simulink model "AcasXuClosedLoop_all_2017b"  (Simulink 9.0)
settings: Solver=VariableStepAuto  StartTime=0.0  StopTime=20  RelTol=1e-3
libraries: neural x14040, simulink x2
(part 16 of 534)

== system AcasXuClosedLoop_all_2017b/networks/Networks_m_3/AcasXu_4_3/Layer 5 ==
  "a{4} " : Inport  PortDimensions=50
      mask Display: e = 0.06;c1 = [120 230 180]/255;c2 = ([120 230 180]+0)/(256+128);c3 = ([120 230 180]+128)/(256+128);patch([0 1 1 0],[0 0 1 1],c1);patch([0 1 1 1-e 1-e 0+e],[0 0 1 1-e 0+e 0+e],c2);patch([0 0 1 1-e 0+e 0+e],[0 1 1 1-e 1-e 0+e],c3);plot([0 1 1 0 0],[0 0 1 1 0]);
  Delays 1 : Subsystem
      mask Display: e = 0.06;c1 = [120 150 230]/255;c2 = ([120 150 230]+0)/383;c3 = ([120 150 230]+128)/383;patch([0 1 1 0],[0 0 1 1],c1);patch([0 1 1 1-e 1-e 0+e],[0 0 1 1-e 0+e 0+e],c2);patch([0 0 1 1-e 0+e 0+e],[0 1 1 1-e 1-e 0+e],c3);plot([0 1 1 0 0],[0 0 1 1 0]);disp('0');
  "LW{5,4}" : Subsystem
      mask Display: e = 0.06;c1 = [120 150 230]/255;c2 = ([120 150 230]+0)/383;c3 = ([120 150 230]+128)/383;patch([0 1 1 0],[0 0 1 1],c1);patch([0 1 1 1-e 1-e 0+e],[0 0 1 1-e 0+e 0+e],c2);patch([0 0 1 1-e 0+e 0+e],[0 1 1 1-e 1-e 0+e],c3);plot([0 1 1 0 0],[0 0 1 1 0]);disp('W');
  "b{5}" : Constant  Value=[2.711069999999999868833810978685505688190460205078125;0.373777000000000025892177291098050773143768310546875;0.57107300000000005280043069433304481208324432373046875;0.33265299999999997648814087369828484952449798583984375;-1.344160000000000021458390619955025613307952880859375;-2.043769999999999864570554564124904572963714599609375;0.38722800000000001663380544414394535124301910400390625;-2.6194199999999998595967554138042032718658447265625;1.088109999999999910613723841379396617412567138671875;-1.0533799999999999830180286153336055576801300048828125;-0.33796199999999998464517148022423498332500457763671875;0.9890689999999999759694446765934117138385772705078125;1.7318000000000000060396132539608515799045562744140625;-2.3428100000000000591171556152403354644775390625;0.63078599999999995784349948735325597226619720458984375;0.286934000000000022367885321727953851222991943359375;1.1716200000000001057998133546789176762104034423828125;-1.4532000000000000472510919280466623604297637939453125;1.1512299999999999755573298898525536060333251953125;0.352835999999999982978948764866800047457218170166015625;0.69320499999999996010302538707037456333637237548828125;0.9155189999999999717061882620328105986118316650390625;-0.073791200000000001235633817486814223229885101318359375;-0.701424999999999965183405947755090892314910888671875;-0.0099617400000000001780708913656781078316271305084228515625;0.67791400000000001657696202528313733637332916259765625;-0.375217999999999995974775401919032447040081024169921875;-3.0377700000000000812860889709554612636566162109375;-1.4288600000000000189714910447946749627590179443359375;-3.927169999999999827622332304599694907665252685546875;1.66561000000000003495870259939692914485931396484375;0.90458000000000005069722419648314826190471649169921875;0.284909000000000023344881583398091606795787811279296875;0.034817300000000002413447219851150293834507465362548828125;1.1870600000000000040500935938325710594654083251953125;-2.08082000000000011397105481592006981372833251953125;0.1708320000000000116191500865170382894575595855712890625;2.019769999999999843254272491321898996829986572265625;-1.8944199999999999928235183688229881227016448974609375;-0.045120100000000003259703618141429615207016468048095703125;1.4280699999999999505462255910970270633697509765625;0.7461349999999999926814098216709680855274200439453125;-1.094349999999999933919525574310682713985443115234375;-0.95453500000000002234656903965515084564685821533203125;0.27985599999999999365485336966230534017086029052734375;-1.14261999999999996902033672085963189601898193359375;0.34124100000000001653432946113753132522106170654296875;-1.4796300000000000007815970093361102044582366943359375;-0.210944999999999993622878946553100831806659698486328125;-1.10081000000000006622258297284133732318878173828125]
      mask Display: e = 0.06;c1 = [120 150 230]/255;c2 = ([120 150 230]+0)/383;c3 = ([120 150 230]+128)/383;patch([0 1 1 0],[0 0 1 1],c1);patch([0 1 1 1-e 1-e 0+e],[0 0 1 1-e 0+e 0+e],c2);patch([0 0 1 1-e 0+e 0+e],[0 1 1 1-e 1-e 0+e],c3);plot([0 1 1 0 0],[0 0 1 1 0]);disp('b');
  netsum : Sum  Inputs=2  SaturateOnIntegerOverflow=on  InputSameDT=on  OutDataTypeStr=Inherit: Same as first input
      mask Display: e = 0.06;c1 = [120 150 230]/255;c2 = ([120 150 230]+0)/383;c3 = ([120 150 230]+128)/383;patch([0 1 1 0],[0 0 1 1],c1);patch([0 1 1 1-e 1-e 0+e],[0 0 1 1-e 0+e 0+e],c2);patch([0 0 1 1-e 0+e 0+e],[0 1 1 1-e 1-e 0+e],c3);plot([0 1 1 0 0],[0 0 1 1 0]);disp('+');
  poslin : neural/Transfer Functions/poslin
      settings: SourceProductName=Deep Learning Toolbox  SourceProductBaseCode=NN
  "a{5}" : Outport  InitialOutput=[0;0;0;0;0;0;0;0;0;0;0;0;0;0;0;0;0;0;0;0;0;0;0;0;0;0;0;0;0;0;0;0;0;0;0;0;0;0;0;0;0;0;0;0;0;0;0;0;0;0]
      mask Display: e = 0.06;c1 = [120 230 180]/255;c2 = ([120 230 180]+0)/(256+128);c3 = ([120 230 180]+128)/(256+128);patch([0 1 1 0],[0 0 1 1],c1);patch([0 1 1 1-e 1-e 0+e],[0 0 1 1-e 0+e 0+e],c2);patch([0 0 1 1-e 0+e 0+e],[0 1 1 1-e 1-e 0+e],c3);plot([0 1 1 0 0],[0 0 1 1 0]);
  wires:
  poslin/1 -> "a{5}"/1
  netsum/1 -> poslin/1
  "b{5}"/1 -> netsum/2
  "LW{5,4}"/1("lz{5,4}") -> netsum/1
  Delays 1/1("pd{5,4}") -> "LW{5,4}"/1("ad{5,4}")
  "a{4} "/1 -> Delays 1/1("p{5}")

== system AcasXuClosedLoop_all_2017b/networks/Networks_m_3/AcasXu_4_3/Layer 6 ==
  "a{5} " : Inport  PortDimensions=50
      mask Display: e = 0.06;c1 = [120 230 180]/255;c2 = ([120 230 180]+0)/(256+128);c3 = ([120 230 180]+128)/(256+128);patch([0 1 1 0],[0 0 1 1],c1);patch([0 1 1 1-e 1-e 0+e],[0 0 1 1-e 0+e 0+e],c2);patch([0 0 1 1-e 0+e 0+e],[0 1 1 1-e 1-e 0+e],c3);plot([0 1 1 0 0],[0 0 1 1 0]);
  Delays 1 : Subsystem
      mask Display: e = 0.06;c1 = [120 150 230]/255;c2 = ([120 150 230]+0)/383;c3 = ([120 150 230]+128)/383;patch([0 1 1 0],[0 0 1 1],c1);patch([0 1 1 1-e 1-e 0+e],[0 0 1 1-e 0+e 0+e],c2);patch([0 0 1 1-e 0+e 0+e],[0 1 1 1-e 1-e 0+e],c3);plot([0 1 1 0 0],[0 0 1 1 0]);disp('0');
  "LW{6,5}" : Subsystem
      mask Display: e = 0.06;c1 = [120 150 230]/255;c2 = ([120 150 230]+0)/383;c3 = ([120 150 230]+128)/383;patch([0 1 1 0],[0 0 1 1],c1);patch([0 1 1 1-e 1-e 0+e],[0 0 1 1-e 0+e 0+e],c2);patch([0 0 1 1-e 0+e 0+e],[0 1 1 1-e 1-e 0+e],c3);plot([0 1 1 0 0],[0 0 1 1 0]);disp('W');
  "b{6}" : Constant  Value=[0.390301999999999982282616883821901865303516387939453125;0.03514219999999999843875997385111986659467220306396484375;0.48808499999999999108268866621074266731739044189453125;-0.78509200000000001207212108056410215795040130615234375;0.40505000000000002113864638886298052966594696044921875;0.354125999999999996337152197156683541834354400634765625;-2.48092999999999985760723575367592275142669677734375;0.48545300000000002338396143386489711701869964599609375;0.06425619999999999942819073339705937542021274566650390625;-0.66102099999999996970956317454692907631397247314453125;0.00843759000000000002061906201333840726874768733978271484375;-0.0248394000000000010619505275144547340460121631622314453125;0.278932000000000013262280162962269969284534454345703125;-0.11266500000000000125677246387567720375955104827880859375;0.5741709999999999869402245167293585836887359619140625;0.036816700000000000814193157339104800485074520111083984375;0.35369200000000000638777919448330067098140716552734375;1.1549300000000000121502807814977131783962249755859375;0.2562780000000000057980287238024175167083740234375;0.457390000000000018776091792460647411644458770751953125;0.10435600000000000431299440606380812823772430419921875;0.57056099999999998484412344623706303536891937255859375;0.8967899999999999760547098048846237361431121826171875;-0.03158829999999999971205255633321939967572689056396484375;-0.62138700000000002265920784338959492743015289306640625;0.385780000000000011795009413617663085460662841796875;0.234605000000000007975842208907124586403369903564453125;1.011810000000000098197006082045845687389373779296875;-0.169279000000000012793321957360603846609592437744140625;0.2365170000000000050111026439481065608561038970947265625;-0.4282369999999999787831939102034084498882293701171875;-0.55425899999999994616217691145720891654491424560546875;0.354545999999999972285280591677292250096797943115234375;-0.01203309999999999969910735586609007441438734531402587890625;0.61969700000000005335465402822592295706272125244140625;0.279428999999999982950527055436396040022373199462890625;-1.2366299999999998959765434847213327884674072265625;-0.36774099999999998455990635193302296102046966552734375;0.1561099999999999987654319966168259270489215850830078125;-0.256284000000000011798562127296463586390018463134765625;-0.01161180000000000024640289808530724258162081241607666015625;0.42765500000000000735411731511703692376613616943359375;-0.281951000000000007172928917498211376368999481201171875;0.33602100000000001411848415955319069325923919677734375;0.050025199999999998834443459827525657601654529571533203125;0.223339000000000009737988193592173047363758087158203125;0.4687769999999999992468247000942938029766082763671875;-1.3342400000000000925837184695410542190074920654296875;0.1654650000000000009681144774731365032494068145751953125;0.194346999999999991981525226947269402444362640380859375]
      mask Display: e = 0.06;c1 = [120 150 230]/255;c2 = ([120 150 230]+0)/383;c3 = ([120 150 230]+128)/383;patch([0 1 1 0],[0 0 1 1],c1);patch([0 1 1 1-e 1-e 0+e],[0 0 1 1-e 0+e 0+e],c2);patch([0 0 1 1-e 0+e 0+e],[0 1 1 1-e 1-e 0+e],c3);plot([0 1 1 0 0],[0 0 1 1 0]);disp('b');
  netsum : Sum  Inputs=2  SaturateOnIntegerOverflow=on  InputSameDT=on  OutDataTypeStr=Inherit: Same as first input
      mask Display: e = 0.06;c1 = [120 150 230]/255;c2 = ([120 150 230]+0)/383;c3 = ([120 150 230]+128)/383;patch([0 1 1 0],[0 0 1 1],c1);patch([0 1 1 1-e 1-e 0+e],[0 0 1 1-e 0+e 0+e],c2);patch([0 0 1 1-e 0+e 0+e],[0 1 1 1-e 1-e 0+e],c3);plot([0 1 1 0 0],[0 0 1 1 0]);disp('+');
  poslin : neural/Transfer Functions/poslin
      settings: SourceProductName=Deep Learning Toolbox  SourceProductBaseCode=NN
  "a{6}" : Outport  InitialOutput=[0;0;0;0;0;0;0;0;0;0;0;0;0;0;0;0;0;0;0;0;0;0;0;0;0;0;0;0;0;0;0;0;0;0;0;0;0;0;0;0;0;0;0;0;0;0;0;0;0;0]
      mask Display: e = 0.06;c1 = [120 230 180]/255;c2 = ([120 230 180]+0)/(256+128);c3 = ([120 230 180]+128)/(256+128);patch([0 1 1 0],[0 0 1 1],c1);patch([0 1 1 1-e 1-e 0+e],[0 0 1 1-e 0+e 0+e],c2);patch([0 0 1 1-e 0+e 0+e],[0 1 1 1-e 1-e 0+e],c3);plot([0 1 1 0 0],[0 0 1 1 0]);
  wires:
  poslin/1 -> "a{6}"/1
  netsum/1 -> poslin/1
  "b{6}"/1 -> netsum/2
  "LW{6,5}"/1("lz{6,5}") -> netsum/1
  Delays 1/1("pd{6,5}") -> "LW{6,5}"/1("ad{6,5}")
  "a{5} "/1 -> Delays 1/1("p{6}")

== system AcasXuClosedLoop_all_2017b/networks/Networks_m_3/AcasXu_4_3/Layer 7 ==
  "a{6} " : Inport  PortDimensions=50
      mask Display: e = 0.06;c1 = [120 230 180]/255;c2 = ([120 230 180]+0)/(256+128);c3 = ([120 230 180]+128)/(256+128);patch([0 1 1 0],[0 0 1 1],c1);patch([0 1 1 1-e 1-e 0+e],[0 0 1 1-e 0+e 0+e],c2);patch([0 0 1 1-e 0+e 0+e],[0 1 1 1-e 1-e 0+e],c3);plot([0 1 1 0 0],[0 0 1 1 0]);
  Delays 1 : Subsystem
      mask Display: e = 0.06;c1 = [120 150 230]/255;c2 = ([120 150 230]+0)/383;c3 = ([120 150 230]+128)/383;patch([0 1 1 0],[0 0 1 1],c1);patch([0 1 1 1-e 1-e 0+e],[0 0 1 1-e 0+e 0+e],c2);patch([0 0 1 1-e 0+e 0+e],[0 1 1 1-e 1-e 0+e],c3);plot([0 1 1 0 0],[0 0 1 1 0]);disp('0');
  "LW{7,6}" : Subsystem
      mask Display: e = 0.06;c1 = [120 150 230]/255;c2 = ([120 150 230]+0)/383;c3 = ([120 150 230]+128)/383;patch([0 1 1 0],[0 0 1 1],c1);patch([0 1 1 1-e 1-e 0+e],[0 0 1 1-e 0+e 0+e],c2);patch([0 0 1 1-e 0+e 0+e],[0 1 1 1-e 1-e 0+e],c3);plot([0 1 1 0 0],[0 0 1 1 0]);disp('W');
  "b{7}" : Constant  Value=[-0.0204165000000000006197264923457623808644711971282958984375;-0.019061399999999999177457965515714022330939769744873046875;0.01847669999999999868034450400955392979085445404052734375;-0.0185021999999999998964828051839504041709005832672119140625;0.01933920000000000083417717178235761821269989013671875]
      mask Display: e = 0.06;c1 = [120 150 230]/255;c2 = ([120 150 230]+0)/383;c3 = ([120 150 230]+128)/383;patch([0 1 1 0],[0 0 1 1],c1);patch([0 1 1 1-e 1-e 0+e],[0 0 1 1-e 0+e 0+e],c2);patch([0 0 1 1-e 0+e 0+e],[0 1 1 1-e 1-e 0+e],c3);plot([0 1 1 0 0],[0 0 1 1 0]);disp('b');
  netsum : Sum  Inputs=2  SaturateOnIntegerOverflow=on  InputSameDT=on  OutDataTypeStr=Inherit: Same as first input
      mask Display: e = 0.06;c1 = [120 150 230]/255;c2 = ([120 150 230]+0)/383;c3 = ([120 150 230]+128)/383;patch([0 1 1 0],[0 0 1 1],c1);patch([0 1 1 1-e 1-e 0+e],[0 0 1 1-e 0+e 0+e],c2);patch([0 0 1 1-e 0+e 0+e],[0 1 1 1-e 1-e 0+e],c3);plot([0 1 1 0 0],[0 0 1 1 0]);disp('+');
  purelin : neural/Transfer Functions/purelin
      settings: SourceProductName=Deep Learning Toolbox
  "a{7}" : Outport  InitialOutput=[0;0;0;0;0]
      mask Display: e = 0.06;c1 = [120 230 180]/255;c2 = ([120 230 180]+0)/(256+128);c3 = ([120 230 180]+128)/(256+128);patch([0 1 1 0],[0 0 1 1],c1);patch([0 1 1 1-e 1-e 0+e],[0 0 1 1-e 0+e 0+e],c2);patch([0 0 1 1-e 0+e 0+e],[0 1 1 1-e 1-e 0+e],c3);plot([0 1 1 0 0],[0 0 1 1 0]);
  wires:
  purelin/1 -> "a{7}"/1
  netsum/1 -> purelin/1
  "b{7}"/1 -> netsum/2
  "LW{7,6}"/1("lz{7,6}") -> netsum/1
  Delays 1/1("pd{7,6}") -> "LW{7,6}"/1("ad{7,6}")
  "a{6} "/1 -> Delays 1/1("p{7}")

== system AcasXuClosedLoop_all_2017b/networks/Networks_m_3/AcasXu_4_3/Process Input 1 ==
  x : Inport  PortDimensions=5
      mask Display: e = 0.06;c1 = [120 230 180]/255;c2 = ([120 230 180]+0)/(256+128);c3 = ([120 230 180]+128)/(256+128);patch([0 1 1 0],[0 0 1 1],c1);patch([0 1 1 1-e 1-e 0+e],[0 0 1 1-e 0+e 0+e],c2);patch([0 0 1 1-e 0+e 0+e],[0 1 1 1-e 1-e 0+e],c3);plot([0 1 1 0 0],[0 0 1 1 0]);
  p : Outport  PortDimensions=5
      mask Display: e = 0.06;c1 = [120 230 180]/255;c2 = ([120 230 180]+0)/(256+128);c3 = ([120 230 180]+128)/(256+128);patch([0 1 1 0],[0 0 1 1],c1);patch([0 1 1 1-e 1-e 0+e],[0 0 1 1-e 0+e 0+e],c2);patch([0 0 1 1-e 0+e 0+e],[0 1 1 1-e 1-e 0+e],c3);plot([0 1 1 0 0],[0 0 1 1 0]);
  wires:
  x/1 -> p/1

== system AcasXuClosedLoop_all_2017b/networks/Networks_m_3/AcasXu_4_3/Process Output 1 ==
  a : Inport  PortDimensions=5
      mask Display: e = 0.06;c1 = [120 230 180]/255;c2 = ([120 230 180]+0)/(256+128);c3 = ([120 230 180]+128)/(256+128);patch([0 1 1 0],[0 0 1 1],c1);patch([0 1 1 1-e 1-e 0+e],[0 0 1 1-e 0+e 0+e],c2);patch([0 0 1 1-e 0+e 0+e],[0 1 1 1-e 1-e 0+e],c3);plot([0 1 1 0 0],[0 0 1 1 0]);
  y : Outport  PortDimensions=5
      mask Display: e = 0.06;c1 = [120 230 180]/255;c2 = ([120 230 180]+0)/(256+128);c3 = ([120 230 180]+128)/(256+128);patch([0 1 1 0],[0 0 1 1],c1);patch([0 1 1 1-e 1-e 0+e],[0 0 1 1-e 0+e 0+e],c2);patch([0 0 1 1-e 0+e 0+e],[0 1 1 1-e 1-e 0+e],c3);plot([0 1 1 0 0],[0 0 1 1 0]);
  wires:
  a/1 -> y/1

== system AcasXuClosedLoop_all_2017b/networks/Networks_m_3/AcasXu_5_3/Layer 1 ==
  "p{1}" : Inport  PortDimensions=5
      mask Display: e = 0.06;c1 = [120 230 180]/255;c2 = ([120 230 180]+0)/(256+128);c3 = ([120 230 180]+128)/(256+128);patch([0 1 1 0],[0 0 1 1],c1);patch([0 1 1 1-e 1-e 0+e],[0 0 1 1-e 0+e 0+e],c2);patch([0 0 1 1-e 0+e 0+e],[0 1 1 1-e 1-e 0+e],c3);plot([0 1 1 0 0],[0 0 1 1 0]);
  Delays 1 : Subsystem
      mask Display: e = 0.06;c1 = [120 150 230]/255;c2 = ([120 150 230]+0)/383;c3 = ([120 150 230]+128)/383;patch([0 1 1 0],[0 0 1 1],c1);patch([0 1 1 1-e 1-e 0+e],[0 0 1 1-e 0+e 0+e],c2);patch([0 0 1 1-e 0+e 0+e],[0 1 1 1-e 1-e 0+e],c3);plot([0 1 1 0 0],[0 0 1 1 0]);disp('0');
  "IW{1,1}" : Subsystem
      mask Display: e = 0.06;c1 = [120 150 230]/255;c2 = ([120 150 230]+0)/383;c3 = ([120 150 230]+128)/383;patch([0 1 1 0],[0 0 1 1],c1);patch([0 1 1 1-e 1-e 0+e],[0 0 1 1-e 0+e 0+e],c2);patch([0 0 1 1-e 0+e 0+e],[0 1 1 1-e 1-e 0+e],c3);plot([0 1 1 0 0],[0 0 1 1 0]);disp('W');
  "b{1}" : Constant  Value=[-0.3715450000000000141398004416259936988353729248046875;0.65812199999999998478728002737625502049922943115234375;-0.2231499999999999872546396773032029159367084503173828125;0.52022299999999999098321268320432864129543304443359375;0.0173735999999999994602983832692189025692641735076904296875;-0.03446559999999999901465486118468106724321842193603515625;0.1578150000000000108268949361445265822112560272216796875;-0.299049999999999982502885131907532922923564910888671875;-0.672749000000000041410430640098638832569122314453125;-0.64948200000000000375877107217092998325824737548828125;-0.50798299999999996234834043207229115068912506103515625;-0.69301800000000002288658151883282698690891265869140625;0.060070400000000002960209855018547386862337589263916015625;-0.12440199999999999869171318778171553276479244232177734375;-0.387757999999999991569410440206411294639110565185546875;-0.007049680000000000008875122858853501384146511554718017578125;0.384906000000000025895730004776851274073123931884765625;-0.060446699999999999042277210037354961968958377838134765625;-0.83811400000000002563638190622441470623016357421875;0.183143000000000000238031816479633562266826629638671875;0.0108234000000000003816058580241588060744106769561767578125;-0.489862999999999992883914501362596638500690460205078125;-0.88090999999999997083222069704788736999034881591796875;0.42101100000000002410160959698259830474853515625;0.000578735000000000034993397068916465286747552454471588134765625;-0.352814000000000016488144183313124813139438629150390625;-0.054441200000000002090505546448184759356081485748291015625;0.016837600000000001176925223944635945372283458709716796875;-0.019340400000000000646505071699721156619489192962646484375;0.2896549999999999958077978590154089033603668212890625;-0.48292000000000001591615728102624416351318359375;-0.5773070000000000145945477925124578177928924560546875;-0.0178104000000000005365929922618306591175496578216552734375;-0.66247000000000000330402372128446586430072784423828125;-0.0340055999999999969407582511848886497318744659423828125;-0.222245999999999999108268866621074266731739044189453125;-0.12642900000000001359268253509071655571460723876953125;-0.420171000000000016694201576683553867042064666748046875;0.235551000000000010370371228418662212789058685302734375;0.0307842000000000011461498417020266060717403888702392578125;0.213629000000000013326229009180678986012935638427734375;-0.0248257999999999985629717258461823803372681140899658203125;0.090437500000000003996802888650563545525074005126953125;0.251244999999999996109778521713451482355594635009765625;0.305526000000000019785062477239989675581455230712890625;-0.12407100000000000072919448257380281575024127960205078125;-0.0225280999999999988203658318752786726690828800201416015625;-0.2296080000000000065352168121535214595496654510498046875;0.12387600000000000000088817841970012523233890533447265625;0.1450490000000000112567732912793871946632862091064453125]
      mask Display: e = 0.06;c1 = [120 150 230]/255;c2 = ([120 150 230]+0)/383;c3 = ([120 150 230]+128)/383;patch([0 1 1 0],[0 0 1 1],c1);patch([0 1 1 1-e 1-e 0+e],[0 0 1 1-e 0+e 0+e],c2);patch([0 0 1 1-e 0+e 0+e],[0 1 1 1-e 1-e 0+e],c3);plot([0 1 1 0 0],[0 0 1 1 0]);disp('b');
  netsum : Sum  Inputs=2  SaturateOnIntegerOverflow=on  InputSameDT=on  OutDataTypeStr=Inherit: Same as first input
      mask Display: e = 0.06;c1 = [120 150 230]/255;c2 = ([120 150 230]+0)/383;c3 = ([120 150 230]+128)/383;patch([0 1 1 0],[0 0 1 1],c1);patch([0 1 1 1-e 1-e 0+e],[0 0 1 1-e 0+e 0+e],c2);patch([0 0 1 1-e 0+e 0+e],[0 1 1 1-e 1-e 0+e],c3);plot([0 1 1 0 0],[0 0 1 1 0]);disp('+');
  poslin : neural/Transfer Functions/poslin
      settings: SourceProductName=Deep Learning Toolbox  SourceProductBaseCode=NN
  "a{1}" : Outport  InitialOutput=[0;0;0;0;0;0;0;0;0;0;0;0;0;0;0;0;0;0;0;0;0;0;0;0;0;0;0;0;0;0;0;0;0;0;0;0;0;0;0;0;0;0;0;0;0;0;0;0;0;0]
      mask Display: e = 0.06;c1 = [120 230 180]/255;c2 = ([120 230 180]+0)/(256+128);c3 = ([120 230 180]+128)/(256+128);patch([0 1 1 0],[0 0 1 1],c1);patch([0 1 1 1-e 1-e 0+e],[0 0 1 1-e 0+e 0+e],c2);patch([0 0 1 1-e 0+e 0+e],[0 1 1 1-e 1-e 0+e],c3);plot([0 1 1 0 0],[0 0 1 1 0]);
  wires:
  poslin/1 -> "a{1}"/1
  netsum/1 -> poslin/1
  "b{1}"/1 -> netsum/2
  "IW{1,1}"/1("iz{1,1}") -> netsum/1
  Delays 1/1("pd{1,1}") -> "IW{1,1}"/1("pd{1,1}")
  "p{1}"/1 -> Delays 1/1("p{1}")

== system AcasXuClosedLoop_all_2017b/networks/Networks_m_3/AcasXu_5_3/Layer 2 ==
  "a{1} " : Inport  PortDimensions=50
      mask Display: e = 0.06;c1 = [120 230 180]/255;c2 = ([120 230 180]+0)/(256+128);c3 = ([120 230 180]+128)/(256+128);patch([0 1 1 0],[0 0 1 1],c1);patch([0 1 1 1-e 1-e 0+e],[0 0 1 1-e 0+e 0+e],c2);patch([0 0 1 1-e 0+e 0+e],[0 1 1 1-e 1-e 0+e],c3);plot([0 1 1 0 0],[0 0 1 1 0]);
  Delays 1 : Subsystem
      mask Display: e = 0.06;c1 = [120 150 230]/255;c2 = ([120 150 230]+0)/383;c3 = ([120 150 230]+128)/383;patch([0 1 1 0],[0 0 1 1],c1);patch([0 1 1 1-e 1-e 0+e],[0 0 1 1-e 0+e 0+e],c2);patch([0 0 1 1-e 0+e 0+e],[0 1 1 1-e 1-e 0+e],c3);plot([0 1 1 0 0],[0 0 1 1 0]);disp('0');
  "LW{2,1}" : Subsystem
      mask Display: e = 0.06;c1 = [120 150 230]/255;c2 = ([120 150 230]+0)/383;c3 = ([120 150 230]+128)/383;patch([0 1 1 0],[0 0 1 1],c1);patch([0 1 1 1-e 1-e 0+e],[0 0 1 1-e 0+e 0+e],c2);patch([0 0 1 1-e 0+e 0+e],[0 1 1 1-e 1-e 0+e],c3);plot([0 1 1 0 0],[0 0 1 1 0]);disp('W');
  "b{2}" : Constant  Value=[-0.1676829999999999987192467187924194149672985076904296875;0.310601999999999989210408557482878677546977996826171875;-0.96966600000000002790301323329913429915904998779296875;-0.041570500000000003282263350001812796108424663543701171875;-0.481177000000000021362467350627412088215351104736328125;-0.65698599999999995890931359099340625107288360595703125;-0.06350719999999999976214581920430646277964115142822265625;0.0496308000000000026918911544271395541727542877197265625;1.4830300000000000704147851138259284198284149169921875;-0.347907000000000021788792992083472199738025665283203125;-0.007282730000000000279702927485914187855087220668792724609375;0.3084700000000000219557705349870957434177398681640625;1.3338699999999998890842789478483609855175018310546875;0.4662450000000000205346850634668953716754913330078125;-0.01525540000000000055713211821739605511538684368133544921875;-0.50505999999999995342392367092543281614780426025390625;-0.43938500000000002554401135057560168206691741943359375;-0.73899400000000003974065521106240339577198028564453125;0.65507499999999996287414205653476528823375701904296875;-0.164113000000000008871126055964850820600986480712890625;-0.05797120000000000050732751333271153271198272705078125;1.4484500000000000152766688188421539962291717529296875;0.020181100000000000427302637717730249278247356414794921875;-0.4089059999999999917008608463220298290252685546875;-0.37971100000000002072653160212212242186069488525390625;-0.70986000000000004650502205549855716526508331298828125;-0.95234399999999996833111026717233471572399139404296875;-0.017702099999999998392041788974893279373645782470703125;-1.8702300000000000590461013416643254458904266357421875;-0.00840982000000000014250378654878659290261566638946533203125;0.0224244000000000005823341808763871085830032825469970703125;-0.6815130000000000354276608049985952675342559814453125;-0.5405290000000000372182284991140477359294891357421875;0.1988499999999999989785948173448559828102588653564453125;0.03694530000000000036219915955371106974780559539794921875;-0.492931999999999981287857053757761605083942413330078125;-0.01329559999999999943265382995605250471271574497222900390625;-0.0114491000000000002490008199629301088862121105194091796875;0.89915599999999995528554563861689530313014984130859375;-0.159463999999999994638955058690044097602367401123046875;-1.50035000000000007247535904753021895885467529296875;0.57026699999999996837374283131794072687625885009765625;-1.3336099999999999621280721839866600930690765380859375;-0.27888400000000002076916416626772843301296234130859375;-0.40673999999999999044320020402665250003337860107421875;-0.75057099999999998818367430430953390896320343017578125;0.62766299999999997094590753476950339972972869873046875;0.558486999999999955690554998000152409076690673828125;-0.0251526000000000006517897332969369017519056797027587890625;-0.060903300000000000491429119620079291053116321563720703125]
      mask Display: e = 0.06;c1 = [120 150 230]/255;c2 = ([120 150 230]+0)/383;c3 = ([120 150 230]+128)/383;patch([0 1 1 0],[0 0 1 1],c1);patch([0 1 1 1-e 1-e 0+e],[0 0 1 1-e 0+e 0+e],c2);patch([0 0 1 1-e 0+e 0+e],[0 1 1 1-e 1-e 0+e],c3);plot([0 1 1 0 0],[0 0 1 1 0]);disp('b');
  netsum : Sum  Inputs=2  SaturateOnIntegerOverflow=on  InputSameDT=on  OutDataTypeStr=Inherit: Same as first input
      mask Display: e = 0.06;c1 = [120 150 230]/255;c2 = ([120 150 230]+0)/383;c3 = ([120 150 230]+128)/383;patch([0 1 1 0],[0 0 1 1],c1);patch([0 1 1 1-e 1-e 0+e],[0 0 1 1-e 0+e 0+e],c2);patch([0 0 1 1-e 0+e 0+e],[0 1 1 1-e 1-e 0+e],c3);plot([0 1 1 0 0],[0 0 1 1 0]);disp('+');
  poslin : neural/Transfer Functions/poslin
      settings: SourceProductName=Deep Learning Toolbox  SourceProductBaseCode=NN
  "a{2}" : Outport  InitialOutput=[0;0;0;0;0;0;0;0;0;0;0;0;0;0;0;0;0;0;0;0;0;0;0;0;0;0;0;0;0;0;0;0;0;0;0;0;0;0;0;0;0;0;0;0;0;0;0;0;0;0]
      mask Display: e = 0.06;c1 = [120 230 180]/255;c2 = ([120 230 180]+0)/(256+128);c3 = ([120 230 180]+128)/(256+128);patch([0 1 1 0],[0 0 1 1],c1);patch([0 1 1 1-e 1-e 0+e],[0 0 1 1-e 0+e 0+e],c2);patch([0 0 1 1-e 0+e 0+e],[0 1 1 1-e 1-e 0+e],c3);plot([0 1 1 0 0],[0 0 1 1 0]);
  wires:
  poslin/1 -> "a{2}"/1
  netsum/1 -> poslin/1
  "b{2}"/1 -> netsum/2
  "LW{2,1}"/1("lz{2,1}") -> netsum/1
  Delays 1/1("pd{2,1}") -> "LW{2,1}"/1("ad{2,1}")
  "a{1} "/1 -> Delays 1/1("p{2}")

== system AcasXuClosedLoop_all_2017b/networks/Networks_m_3/AcasXu_5_3/Layer 3 ==
  "a{2} " : Inport  PortDimensions=50
      mask Display: e = 0.06;c1 = [120 230 180]/255;c2 = ([120 230 180]+0)/(256+128);c3 = ([120 230 180]+128)/(256+128);patch([0 1 1 0],[0 0 1 1],c1);patch([0 1 1 1-e 1-e 0+e],[0 0 1 1-e 0+e 0+e],c2);patch([0 0 1 1-e 0+e 0+e],[0 1 1 1-e 1-e 0+e],c3);plot([0 1 1 0 0],[0 0 1 1 0]);
  Delays 1 : Subsystem
      mask Display: e = 0.06;c1 = [120 150 230]/255;c2 = ([120 150 230]+0)/383;c3 = ([120 150 230]+128)/383;patch([0 1 1 0],[0 0 1 1],c1);patch([0 1 1 1-e 1-e 0+e],[0 0 1 1-e 0+e 0+e],c2);patch([0 0 1 1-e 0+e 0+e],[0 1 1 1-e 1-e 0+e],c3);plot([0 1 1 0 0],[0 0 1 1 0]);disp('0');
  "LW{3,2}" : Subsystem
      mask Display: e = 0.06;c1 = [120 150 230]/255;c2 = ([120 150 230]+0)/383;c3 = ([120 150 230]+128)/383;patch([0 1 1 0],[0 0 1 1],c1);patch([0 1 1 1-e 1-e 0+e],[0 0 1 1-e 0+e 0+e],c2);patch([0 0 1 1-e 0+e 0+e],[0 1 1 1-e 1-e 0+e],c3);plot([0 1 1 0 0],[0 0 1 1 0]);disp('W');
  "b{3}" : Constant  Value=[0.1510060000000000013375967000683885999023914337158203125;0.78885499999999997289279463075217790901660919189453125;-1.1981900000000000883204620549804531037807464599609375;-0.588840999999999947789319776347838342189788818359375;-0.397129000000000009773515330380178056657314300537109375;-0.280633999999999994567900785114034079015254974365234375;1.6851499999999999257482841130695305764675140380859375;-0.6019039999999999945856643535080365836620330810546875;-1.6417800000000000171240799318184144794940948486328125;-2.115330000000000154614099301397800445556640625;-0.0430941999999999991732835269431234337389469146728515625;-0.75815500000000002334132886971929110586643218994140625;-0.0785547000000000050778936611095559783279895782470703125;1.3500199999999999977973175191436894237995147705078125;-1.826869999999999993889332472463138401508331298828125;-1.043509999999999937614347800263203680515289306640625;-0.551185999999999953757878756732679903507232666015625;-2.163539999999999796642669025459326803684234619140625;-0.84310300000000004683187171394820325076580047607421875;-0.2149149999999999949285012235122849233448505401611328125;-0.8115930000000000088533624875708483159542083740234375;0.55653099999999999791810978422290645539760589599609375;0.55281100000000005234568334344658069312572479248046875;0.106677999999999995051069845430902205407619476318359375;0.48474899999999998545519019899074919521808624267578125;0.34542499999999998205879592205747030675411224365234375;1.12647999999999992581933838664554059505462646484375;-1.0468900000000000982680603556218557059764862060546875;-1.76700999999999996958877090946771204471588134765625;-0.141981999999999997097432924419990740716457366943359375;0.81875200000000003530686853991937823593616485595703125;-1.427729999999999943582906780648045241832733154296875;-0.88332200000000005157829718882567249238491058349609375;-0.58885799999999999254640670187654905021190643310546875;1.00330999999999992411403582082130014896392822265625;0.323604000000000002756905814749188721179962158203125;-2.16692999999999980076381689286790788173675537109375;0.34770800000000001706013108560000546276569366455078125;-0.61344100000000001404742988597718067467212677001953125;-3.37966999999999995196731106261722743511199951171875;-0.318205000000000015614176618328201584517955780029296875;-0.065882300000000004747136017613229341804981231689453125;0.2342640000000000000124344978758017532527446746826171875;-1.055919999999999969730879456619732081890106201171875;0.7741190000000000015489831639570184051990509033203125;0.44780399999999997984190258648595772683620452880859375;-0.554389000000000020662582755903713405132293701171875;-0.83642099999999997006483454242697916924953460693359375;1.2263699999999999601385525238583795726299285888671875;0.2497109999999999885300638879925827495753765106201171875]
      mask Display: e = 0.06;c1 = [120 150 230]/255;c2 = ([120 150 230]+0)/383;c3 = ([120 150 230]+128)/383;patch([0 1 1 0],[0 0 1 1],c1);patch([0 1 1 1-e 1-e 0+e],[0 0 1 1-e 0+e 0+e],c2);patch([0 0 1 1-e 0+e 0+e],[0 1 1 1-e 1-e 0+e],c3);plot([0 1 1 0 0],[0 0 1 1 0]);disp('b');
  netsum : Sum  Inputs=2  SaturateOnIntegerOverflow=on  InputSameDT=on  OutDataTypeStr=Inherit: Same as first input
      mask Display: e = 0.06;c1 = [120 150 230]/255;c2 = ([120 150 230]+0)/383;c3 = ([120 150 230]+128)/383;patch([0 1 1 0],[0 0 1 1],c1);patch([0 1 1 1-e 1-e 0+e],[0 0 1 1-e 0+e 0+e],c2);patch([0 0 1 1-e 0+e 0+e],[0 1 1 1-e 1-e 0+e],c3);plot([0 1 1 0 0],[0 0 1 1 0]);disp('+');
  poslin : neural/Transfer Functions/poslin
      settings: SourceProductName=Deep Learning Toolbox  SourceProductBaseCode=NN
  "a{3}" : Outport  InitialOutput=[0;0;0;0;0;0;0;0;0;0;0;0;0;0;0;0;0;0;0;0;0;0;0;0;0;0;0;0;0;0;0;0;0;0;0;0;0;0;0;0;0;0;0;0;0;0;0;0;0;0]
      mask Display: e = 0.06;c1 = [120 230 180]/255;c2 = ([120 230 180]+0)/(256+128);c3 = ([120 230 180]+128)/(256+128);patch([0 1 1 0],[0 0 1 1],c1);patch([0 1 1 1-e 1-e 0+e],[0 0 1 1-e 0+e 0+e],c2);patch([0 0 1 1-e 0+e 0+e],[0 1 1 1-e 1-e 0+e],c3);plot([0 1 1 0 0],[0 0 1 1 0]);
  wires:
  poslin/1 -> "a{3}"/1
  netsum/1 -> poslin/1
  "b{3}"/1 -> netsum/2
  "LW{3,2}"/1("lz{3,2}") -> netsum/1
  Delays 1/1("pd{3,2}") -> "LW{3,2}"/1("ad{3,2}")
  "a{2} "/1 -> Delays 1/1("p{3}")

== system AcasXuClosedLoop_all_2017b/networks/Networks_m_3/AcasXu_5_3/Layer 4 ==
  "a{3} " : Inport  PortDimensions=50
      mask Display: e = 0.06;c1 = [120 230 180]/255;c2 = ([120 230 180]+0)/(256+128);c3 = ([120 230 180]+128)/(256+128);patch([0 1 1 0],[0 0 1 1],c1);patch([0 1 1 1-e 1-e 0+e],[0 0 1 1-e 0+e 0+e],c2);patch([0 0 1 1-e 0+e 0+e],[0 1 1 1-e 1-e 0+e],c3);plot([0 1 1 0 0],[0 0 1 1 0]);
  Delays 1 : Subsystem
      mask Display: e = 0.06;c1 = [120 150 230]/255;c2 = ([120 150 230]+0)/383;c3 = ([120 150 230]+128)/383;patch([0 1 1 0],[0 0 1 1],c1);patch([0 1 1 1-e 1-e 0+e],[0 0 1 1-e 0+e 0+e],c2);patch([0 0 1 1-e 0+e 0+e],[0 1 1 1-e 1-e 0+e],c3);plot([0 1 1 0 0],[0 0 1 1 0]);disp('0');
  "LW{4,3}" : Subsystem
      mask Display: e = 0.06;c1 = [120 150 230]/255;c2 = ([120 150 230]+0)/383;c3 = ([120 150 230]+128)/383;patch([0 1 1 0],[0 0 1 1],c1);patch([0 1 1 1-e 1-e 0+e],[0 0 1 1-e 0+e 0+e],c2);patch([0 0 1 1-e 0+e 0+e],[0 1 1 1-e 1-e 0+e],c3);plot([0 1 1 0 0],[0 0 1 1 0]);disp('W');
  "b{4}" : Constant  Value=[0.7212579999999999547100060226512141525745391845703125;0.54630999999999996230570786792668513953685760498046875;1.1140399999999999192112909440766088664531707763671875;-0.442867999999999983895548893997329287230968475341796875;0.3276339999999999808011352797620929777622222900390625;0.08699379999999999613091716810231446288526058197021484375;-0.95715300000000003155520289510604925453662872314453125;0.2160539999999999960511587460132432170212268829345703125;-4.0011200000000002319211489520967006683349609375;-0.326593999999999995420552068026154302060604095458984375;-0.652746000000000048402171159978024661540985107421875;-0.023803100000000000646682707383661181665956974029541015625;-0.75029800000000002047073621724848635494709014892578125;0.06980310000000000680842049405327998101711273193359375;-0.1605459999999999942676964792553917504847049713134765625;-0.215036000000000004916955731459893286228179931640625;0.53674999999999994937383007709286175668239593505859375;0.496682000000000012374101743262144736945629119873046875;-1.4020900000000000584776671530562452971935272216796875;0.2827859999999999818243168192566372454166412353515625;0.305470999999999992535748560840147547423839569091796875;-0.70448699999999997434230181170278228819370269775390625;-0.80505300000000001858779796748422086238861083984375;1.288419999999999898676605880609713494777679443359375;0.2052150000000000085176310449242009781301021575927734375;-0.6115939999999999709956455262727104127407073974609375;-0.19087799999999999212008106042048893868923187255859375;-1.2522200000000001107736125049996189773082733154296875;-0.65776000000000001133315663537359796464443206787109375;0.0227152000000000013624212868990071001462638378143310546875;0.78801799999999999624122892782907001674175262451171875;0.99007400000000000961364321483415551483631134033203125;0.09256599999999999550670537473706644959747791290283203125;0.261581000000000007954525926834321580827236175537109375;-0.472055999999999975624831449749763123691082000732421875;-0.410337000000000007293721182577428407967090606689453125;-0.256960000000000021724844145865063183009624481201171875;0.52285400000000004094857786185457371175289154052734375;0.307966000000000017511325722807669080793857574462890625;-2.04220999999999985874410413089208304882049560546875;0.1452279999999999959836571861160336993634700775146484375;0.570680999999999993832489053602330386638641357421875;-1.0596300000000000718358705853461287915706634521484375;-0.64597499999999996589394868351519107818603515625;0.8822870000000000434425828643725253641605377197265625;-0.011801499999999999490629676301978179253637790679931640625;-0.16074800000000000199662508748588152229785919189453125;-0.51775300000000001876543365142424590885639190673828125;-3.15078000000000013613998817163519561290740966796875;0.30289500000000002533084852984757162630558013916015625]
      mask Display: e = 0.06;c1 = [120 150 230]/255;c2 = ([120 150 230]+0)/383;c3 = ([120 150 230]+128)/383;patch([0 1 1 0],[0 0 1 1],c1);patch([0 1 1 1-e 1-e 0+e],[0 0 1 1-e 0+e 0+e],c2);patch([0 0 1 1-e 0+e 0+e],[0 1 1 1-e 1-e 0+e],c3);plot([0 1 1 0 0],[0 0 1 1 0]);disp('b');
  netsum : Sum  Inputs=2  SaturateOnIntegerOverflow=on  InputSameDT=on  OutDataTypeStr=Inherit: Same as first input
      mask Display: e = 0.06;c1 = [120 150 230]/255;c2 = ([120 150 230]+0)/383;c3 = ([120 150 230]+128)/383;patch([0 1 1 0],[0 0 1 1],c1);patch([0 1 1 1-e 1-e 0+e],[0 0 1 1-e 0+e 0+e],c2);patch([0 0 1 1-e 0+e 0+e],[0 1 1 1-e 1-e 0+e],c3);plot([0 1 1 0 0],[0 0 1 1 0]);disp('+');
  poslin : neural/Transfer Functions/poslin
      settings: SourceProductName=Deep Learning Toolbox  SourceProductBaseCode=NN
  "a{4}" : Outport  InitialOutput=[0;0;0;0;0;0;0;0;0;0;0;0;0;0;0;0;0;0;0;0;0;0;0;0;0;0;0;0;0;0;0;0;0;0;0;0;0;0;0;0;0;0;0;0;0;0;0;0;0;0]
      mask Display: e = 0.06;c1 = [120 230 180]/255;c2 = ([120 230 180]+0)/(256+128);c3 = ([120 230 180]+128)/(256+128);patch([0 1 1 0],[0 0 1 1],c1);patch([0 1 1 1-e 1-e 0+e],[0 0 1 1-e 0+e 0+e],c2);patch([0 0 1 1-e 0+e 0+e],[0 1 1 1-e 1-e 0+e],c3);plot([0 1 1 0 0],[0 0 1 1 0]);
  wires:
  poslin/1 -> "a{4}"/1
  netsum/1 -> poslin/1
  "b{4}"/1 -> netsum/2
  "LW{4,3}"/1("lz{4,3}") -> netsum/1
  Delays 1/1("pd{4,3}") -> "LW{4,3}"/1("ad{4,3}")
  "a{3} "/1 -> Delays 1/1("p{4}")

== system AcasXuClosedLoop_all_2017b/networks/Networks_m_3/AcasXu_5_3/Layer 5 ==
  "a{4} " : Inport  PortDimensions=50
      mask Display: e = 0.06;c1 = [120 230 180]/255;c2 = ([120 230 180]+0)/(256+128);c3 = ([120 230 180]+128)/(256+128);patch([0 1 1 0],[0 0 1 1],c1);patch([0 1 1 1-e 1-e 0+e],[0 0 1 1-e 0+e 0+e],c2);patch([0 0 1 1-e 0+e 0+e],[0 1 1 1-e 1-e 0+e],c3);plot([0 1 1 0 0],[0 0 1 1 0]);
  Delays 1 : Subsystem
      mask Display: e = 0.06;c1 = [120 150 230]/255;c2 = ([120 150 230]+0)/383;c3 = ([120 150 230]+128)/383;patch([0 1 1 0],[0 0 1 1],c1);patch([0 1 1 1-e 1-e 0+e],[0 0 1 1-e 0+e 0+e],c2);patch([0 0 1 1-e 0+e 0+e],[0 1 1 1-e 1-e 0+e],c3);plot([0 1 1 0 0],[0 0 1 1 0]);disp('0');
  "LW{5,4}" : Subsystem
      mask Display: e = 0.06;c1 = [120 150 230]/255;c2 = ([120 150 230]+0)/383;c3 = ([120 150 230]+128)/383;patch([0 1 1 0],[0 0 1 1],c1);patch([0 1 1 1-e 1-e 0+e],[0 0 1 1-e 0+e 0+e],c2);patch([0 0 1 1-e 0+e 0+e],[0 1 1 1-e 1-e 0+e],c3);plot([0 1 1 0 0],[0 0 1 1 0]);disp('W');
  "b{5}" : Constant  Value=[0.47258400000000000407140987590537406504154205322265625;1.2492300000000000625988150204648263752460479736328125;-4.52592000000000016513013179064728319644927978515625;-0.6574619999999999908624204181251116096973419189453125;-0.028083400000000001417443939999429858289659023284912109375;-0.2020530000000000103721475852580624632537364959716796875;0.70148200000000004994404889657744206488132476806640625;0.6753489999999999771063130538095720112323760986328125;0.75429000000000001602273869139025919139385223388671875;-0.2459340000000000137081457296517328359186649322509765625;0.0716490999999999933489647219175822101533412933349609375;0.222434999999999993836041767281130887567996978759765625;-0.8294580000000000286064505417016334831714630126953125;-0.31864700000000001356426082566031254827976226806640625;-0.00776489999999999992719157404508223407901823520660400390625;0.945052000000000003154809746774844825267791748046875;-4.44582999999999994855670593096874654293060302734375;1.0560400000000000897415475265006534755229949951171875;1.6571499999999999008792883614660240709781646728515625;-0.8016609999999999569553210676531307399272918701171875;1.576729999999999964899188853451050817966461181640625;1.026149999999999895550217843265272676944732666015625;0.049465900000000000147348799828250776045024394989013671875;1.497759999999999980246911945869214832782745361328125;0.249765999999999988023802188763511367142200469970703125;-1.4057500000000000550670620214077644050121307373046875;-0.97891399999999995085175896747387014329433441162109375;-1.11186000000000007048583938740193843841552734375;0.63749500000000003385736135896877385675907135009765625;0.92279999999999995363708649165346287190914154052734375;0.77215100000000003177547114319168031215667724609375;1.1934899999999999398170302811195142567157745361328125;1.3931199999999999139532746994518674910068511962890625;0.484723999999999988208543300061137415468692779541015625;-1.9544799999999999950972551232553087174892425537109375;1.057889999999999997015720509807579219341278076171875;1.955319999999999946993511912296526134014129638671875;-0.8511889999999999734114908278570510447025299072265625;1.4870300000000000739674987926264293491840362548828125;-0.427566999999999974857445295128854922950267791748046875;0.601442000000000032144953365786932408809661865234375;0.38846999999999998198774164848146028816699981689453125;-1.3176200000000000134292577058658935129642486572265625;0.454407999999999978602005512584582902491092681884765625;-0.0168409999999999983322229724080898449756205081939697265625;-0.57140199999999996549604475148953497409820556640625;-1.84166000000000007474909580196253955364227294921875;1.2264999999999999236166559057892300188541412353515625;0.50610399999999999831601371624856255948543548583984375;-0.775908999999999959840124574839137494564056396484375]
      mask Display: e = 0.06;c1 = [120 150 230]/255;c2 = ([120 150 230]+0)/383;c3 = ([120 150 230]+128)/383;patch([0 1 1 0],[0 0 1 1],c1);patch([0 1 1 1-e 1-e 0+e],[0 0 1 1-e 0+e 0+e],c2);patch([0 0 1 1-e 0+e 0+e],[0 1 1 1-e 1-e 0+e],c3);plot([0 1 1 0 0],[0 0 1 1 0]);disp('b');
  netsum : Sum  Inputs=2  SaturateOnIntegerOverflow=on  InputSameDT=on  OutDataTypeStr=Inherit: Same as first input
      mask Display: e = 0.06;c1 = [120 150 230]/255;c2 = ([120 150 230]+0)/383;c3 = ([120 150 230]+128)/383;patch([0 1 1 0],[0 0 1 1],c1);patch([0 1 1 1-e 1-e 0+e],[0 0 1 1-e 0+e 0+e],c2);patch([0 0 1 1-e 0+e 0+e],[0 1 1 1-e 1-e 0+e],c3);plot([0 1 1 0 0],[0 0 1 1 0]);disp('+');
  poslin : neural/Transfer Functions/poslin
      settings: SourceProductName=Deep Learning Toolbox  SourceProductBaseCode=NN
  "a{5}" : Outport  InitialOutput=[0;0;0;0;0;0;0;0;0;0;0;0;0;0;0;0;0;0;0;0;0;0;0;0;0;0;0;0;0;0;0;0;0;0;0;0;0;0;0;0;0;0;0;0;0;0;0;0;0;0]
      mask Display: e = 0.06;c1 = [120 230 180]/255;c2 = ([120 230 180]+0)/(256+128);c3 = ([120 230 180]+128)/(256+128);patch([0 1 1 0],[0 0 1 1],c1);patch([0 1 1 1-e 1-e 0+e],[0 0 1 1-e 0+e 0+e],c2);patch([0 0 1 1-e 0+e 0+e],[0 1 1 1-e 1-e 0+e],c3);plot([0 1 1 0 0],[0 0 1 1 0]);
  wires:
  poslin/1 -> "a{5}"/1
  netsum/1 -> poslin/1
  "b{5}"/1 -> netsum/2
  "LW{5,4}"/1("lz{5,4}") -> netsum/1
  Delays 1/1("pd{5,4}") -> "LW{5,4}"/1("ad{5,4}")
  "a{4} "/1 -> Delays 1/1("p{5}")

== system AcasXuClosedLoop_all_2017b/networks/Networks_m_3/AcasXu_5_3/Layer 6 ==
  "a{5} " : Inport  PortDimensions=50
      mask Display: e = 0.06;c1 = [120 230 180]/255;c2 = ([120 230 180]+0)/(256+128);c3 = ([120 230 180]+128)/(256+128);patch([0 1 1 0],[0 0 1 1],c1);patch([0 1 1 1-e 1-e 0+e],[0 0 1 1-e 0+e 0+e],c2);patch([0 0 1 1-e 0+e 0+e],[0 1 1 1-e 1-e 0+e],c3);plot([0 1 1 0 0],[0 0 1 1 0]);
  Delays 1 : Subsystem
      mask Display: e = 0.06;c1 = [120 150 230]/255;c2 = ([120 150 230]+0)/383;c3 = ([120 150 230]+128)/383;patch([0 1 1 0],[0 0 1 1],c1);patch([0 1 1 1-e 1-e 0+e],[0 0 1 1-e 0+e 0+e],c2);patch([0 0 1 1-e 0+e 0+e],[0 1 1 1-e 1-e 0+e],c3);plot([0 1 1 0 0],[0 0 1 1 0]);disp('0');
  "LW{6,5}" : Subsystem
      mask Display: e = 0.06;c1 = [120 150 230]/255;c2 = ([120 150 230]+0)/383;c3 = ([120 150 230]+128)/383;patch([0 1 1 0],[0 0 1 1],c1);patch([0 1 1 1-e 1-e 0+e],[0 0 1 1-e 0+e 0+e],c2);patch([0 0 1 1-e 0+e 0+e],[0 1 1 1-e 1-e 0+e],c3);plot([0 1 1 0 0],[0 0 1 1 0]);disp('W');
  "b{6}" : Constant  Value=[0.24485299999999998732391759403981268405914306640625;1.3035699999999998954791635696892626583576202392578125;0.06463059999999999638564673887231037952005863189697265625;0.68099699999999996347099795457324944436550140380859375;0.310184000000000015262457964126951992511749267578125;0.0342837000000000002852829084076802246272563934326171875;0.201763999999999998902211473250645212829113006591796875;0.0027227599999999998504895959428040441707707941532135009765625;0.2120390000000000052526871741065406240522861480712890625;-1.0279499999999999193533994912286289036273956298828125;0.224783000000000010576428621789091266691684722900390625;0.058338599999999997403055118638803833164274692535400390625;-0.6257669999999999621564938934170641005039215087890625;-0.00839885999999999931231453587088253698311746120452880859375;-0.2389159999999999894892965812687180005013942718505859375;-0.495479000000000002756905814749188721179962158203125;-0.019560399999999998621458274783435626886785030364990234375;-0.6981420000000000403161948270280845463275909423828125;-1.53960000000000007958078640513122081756591796875;-0.036735499999999997322586153813972487114369869232177734375;-0.807528000000000023561597117804922163486480712890625;-1.8465599999999999791810978422290645539760589599609375;-0.78101699999999996126831547371693886816501617431640625;-0.65409700000000003949907068090396933257579803466796875;0.1082140000000000046309622803164529614150524139404296875;-2.92194999999999982520648700301535427570343017578125;0.25573800000000002086153472191654145717620849609375;-1.617059999999999941877604214823804795742034912109375;-0.32116800000000000903099817151087336242198944091796875;-1.2529600000000000736832816983223892748355865478515625;-0.71420099999999997475441659844364039599895477294921875;-0.406679999999999985949017400344018824398517608642578125;-0.276156999999999985817566994228400290012359619140625;-0.76702199999999998158983771645580418407917022705078125;-0.61882999999999999118927007657475769519805908203125;-0.07028579999999999550208684695462579838931560516357421875;0.0304981999999999996153743353488607681356370449066162109375;0.2280729999999999979554132778503117151558399200439453125;-0.049308600000000001040145747310816659592092037200927734375;0.0909944000000000030592417488151113502681255340576171875;-0.0539395000000000013340439863895880989730358123779296875;-0.7831550000000000455457893622224219143390655517578125;-0.4604690000000000171809233506792224943637847900390625;0.320039000000000017909229654833325184881687164306640625;-0.98684099999999996821742342945071868598461151123046875;-1.108239999999999891855395617312751710414886474609375;-0.216764000000000012224887768752523697912693023681640625;0.9355949999999999544542106377775780856609344482421875;0.2041669999999999873807610129006206989288330078125;-0.325021999999999977593034827805240638554096221923828125]
      mask Display: e = 0.06;c1 = [120 150 230]/255;c2 = ([120 150 230]+0)/383;c3 = ([120 150 230]+128)/383;patch([0 1 1 0],[0 0 1 1],c1);patch([0 1 1 1-e 1-e 0+e],[0 0 1 1-e 0+e 0+e],c2);patch([0 0 1 1-e 0+e 0+e],[0 1 1 1-e 1-e 0+e],c3);plot([0 1 1 0 0],[0 0 1 1 0]);disp('b');
  netsum : Sum  Inputs=2  SaturateOnIntegerOverflow=on  InputSameDT=on  OutDataTypeStr=Inherit: Same as first input
      mask Display: e = 0.06;c1 = [120 150 230]/255;c2 = ([120 150 230]+0)/383;c3 = ([120 150 230]+128)/383;patch([0 1 1 0],[0 0 1 1],c1);patch([0 1 1 1-e 1-e 0+e],[0 0 1 1-e 0+e 0+e],c2);patch([0 0 1 1-e 0+e 0+e],[0 1 1 1-e 1-e 0+e],c3);plot([0 1 1 0 0],[0 0 1 1 0]);disp('+');
  poslin : neural/Transfer Functions/poslin
      settings: SourceProductName=Deep Learning Toolbox  SourceProductBaseCode=NN
  "a{6}" : Outport  InitialOutput=[0;0;0;0;0;0;0;0;0;0;0;0;0;0;0;0;0;0;0;0;0;0;0;0;0;0;0;0;0;0;0;0;0;0;0;0;0;0;0;0;0;0;0;0;0;0;0;0;0;0]
      mask Display: e = 0.06;c1 = [120 230 180]/255;c2 = ([120 230 180]+0)/(256+128);c3 = ([120 230 180]+128)/(256+128);patch([0 1 1 0],[0 0 1 1],c1);patch([0 1 1 1-e 1-e 0+e],[0 0 1 1-e 0+e 0+e],c2);patch([0 0 1 1-e 0+e 0+e],[0 1 1 1-e 1-e 0+e],c3);plot([0 1 1 0 0],[0 0 1 1 0]);
  wires:
  poslin/1 -> "a{6}"/1
  netsum/1 -> poslin/1
  "b{6}"/1 -> netsum/2
  "LW{6,5}"/1("lz{6,5}") -> netsum/1
  Delays 1/1("pd{6,5}") -> "LW{6,5}"/1("ad{6,5}")
  "a{5} "/1 -> Delays 1/1("p{6}")

== system AcasXuClosedLoop_all_2017b/networks/Networks_m_3/AcasXu_5_3/Layer 7 ==
  "a{6} " : Inport  PortDimensions=50
      mask Display: e = 0.06;c1 = [120 230 180]/255;c2 = ([120 230 180]+0)/(256+128);c3 = ([120 230 180]+128)/(256+128);patch([0 1 1 0],[0 0 1 1],c1);patch([0 1 1 1-e 1-e 0+e],[0 0 1 1-e 0+e 0+e],c2);patch([0 0 1 1-e 0+e 0+e],[0 1 1 1-e 1-e 0+e],c3);plot([0 1 1 0 0],[0 0 1 1 0]);
  Delays 1 : Subsystem
      mask Display: e = 0.06;c1 = [120 150 230]/255;c2 = ([120 150 230]+0)/383;c3 = ([120 150 230]+128)/383;patch([0 1 1 0],[0 0 1 1],c1);patch([0 1 1 1-e 1-e 0+e],[0 0 1 1-e 0+e 0+e],c2);patch([0 0 1 1-e 0+e 0+e],[0 1 1 1-e 1-e 0+e],c3);plot([0 1 1 0 0],[0 0 1 1 0]);disp('0');
  "LW{7,6}" : Subsystem
      mask Display: e = 0.06;c1 = [120 150 230]/255;c2 = ([120 150 230]+0)/383;c3 = ([120 150 230]+128)/383;patch([0 1 1 0],[0 0 1 1],c1);patch([0 1 1 1-e 1-e 0+e],[0 0 1 1-e 0+e 0+e],c2);patch([0 0 1 1-e 0+e 0+e],[0 1 1 1-e 1-e 0+e],c3);plot([0 1 1 0 0],[0 0 1 1 0]);disp('W');
  "b{7}" : Constant  Value=[0.026811700000000000809752265240604174323379993438720703125;0.0258377000000000016377565970060459221713244915008544921875;-0.00943751999999999958979035596939866081811487674713134765625;0.020566899999999999071320644361549057066440582275390625;-0.02147380000000000122017951298403204418718814849853515625]
      mask Display: e = 0.06;c1 = [120 150 230]/255;c2 = ([120 150 230]+0)/383;c3 = ([120 150 230]+128)/383;patch([0 1 1 0],[0 0 1 1],c1);patch([0 1 1 1-e 1-e 0+e],[0 0 1 1-e 0+e 0+e],c2);patch([0 0 1 1-e 0+e 0+e],[0 1 1 1-e 1-e 0+e],c3);plot([0 1 1 0 0],[0 0 1 1 0]);disp('b');
  netsum : Sum  Inputs=2  SaturateOnIntegerOverflow=on  InputSameDT=on  OutDataTypeStr=Inherit: Same as first input
      mask Display: e = 0.06;c1 = [120 150 230]/255;c2 = ([120 150 230]+0)/383;c3 = ([120 150 230]+128)/383;patch([0 1 1 0],[0 0 1 1],c1);patch([0 1 1 1-e 1-e 0+e],[0 0 1 1-e 0+e 0+e],c2);patch([0 0 1 1-e 0+e 0+e],[0 1 1 1-e 1-e 0+e],c3);plot([0 1 1 0 0],[0 0 1 1 0]);disp('+');
  purelin : neural/Transfer Functions/purelin
      settings: SourceProductName=Deep Learning Toolbox
  "a{7}" : Outport  InitialOutput=[0;0;0;0;0]
      mask Display: e = 0.06;c1 = [120 230 180]/255;c2 = ([120 230 180]+0)/(256+128);c3 = ([120 230 180]+128)/(256+128);patch([0 1 1 0],[0 0 1 1],c1);patch([0 1 1 1-e 1-e 0+e],[0 0 1 1-e 0+e 0+e],c2);patch([0 0 1 1-e 0+e 0+e],[0 1 1 1-e 1-e 0+e],c3);plot([0 1 1 0 0],[0 0 1 1 0]);
  wires:
  purelin/1 -> "a{7}"/1
  netsum/1 -> purelin/1
  "b{7}"/1 -> netsum/2
  "LW{7,6}"/1("lz{7,6}") -> netsum/1
  Delays 1/1("pd{7,6}") -> "LW{7,6}"/1("ad{7,6}")
  "a{6} "/1 -> Delays 1/1("p{7}")

== system AcasXuClosedLoop_all_2017b/networks/Networks_m_3/AcasXu_5_3/Process Input 1 ==
  x : Inport  PortDimensions=5
      mask Display: e = 0.06;c1 = [120 230 180]/255;c2 = ([120 230 180]+0)/(256+128);c3 = ([120 230 180]+128)/(256+128);patch([0 1 1 0],[0 0 1 1],c1);patch([0 1 1 1-e 1-e 0+e],[0 0 1 1-e 0+e 0+e],c2);patch([0 0 1 1-e 0+e 0+e],[0 1 1 1-e 1-e 0+e],c3);plot([0 1 1 0 0],[0 0 1 1 0]);
  p : Outport  PortDimensions=5
      mask Display: e = 0.06;c1 = [120 230 180]/255;c2 = ([120 230 180]+0)/(256+128);c3 = ([120 230 180]+128)/(256+128);patch([0 1 1 0],[0 0 1 1],c1);patch([0 1 1 1-e 1-e 0+e],[0 0 1 1-e 0+e 0+e],c2);patch([0 0 1 1-e 0+e 0+e],[0 1 1 1-e 1-e 0+e],c3);plot([0 1 1 0 0],[0 0 1 1 0]);
  wires:
  x/1 -> p/1
